simulink model "TosAeb"  (R2021a)
settings: Solver=FixedStepDiscrete  StartTime=0.0  StopTime=inf  FixedStep=0.01  RelTol=1e-3
undefined symbols (not defined in the file or in its folder): ACCModSts, AccSts1, AEB_ObjectMovingOrientation, AEB_ObjectMovingState, CN_BTCActive, CN_CurrentGearR, CN_CurrentGearR_DHT, CN_PTCActive, CN_VDCActive, CN_VSC_EspOff, CN_VSC_FCW_AEB_Error, CN_VSC_FCW_AEB_NoDispay, CN_VSC_FCW_AEB_PerfmDgdtn, CN_VSC_FCW_AEB_TempUnAvlbl, CN_VSC_VehStandstill, CNaccelAvoidMax, CNaccelAvoidMin, CNbrakePedalPress, CNcomfortStrLeftAccelMax, CNcomfortStrLeftAccelMin, CNcomfortStrRightAccelMax, CNcomfortStrRightAccelMin, CNDrvDoorOpen, CNDrvModeEco, CNDrvModeNorm, CNDrvModeOther, CNDrvModeSport, CNDrvSBRreminder, CNNull, CNobjInvalidID, CNPassDoorOpen, CNPassSBRreminder, CNPEBSAccelNegInf, CNPEBSAccelNull, CNPEBSTimeInf, CNPEBSTimeNull, CNSTOPMAX, CNSTOPNULL, CNsubjWidth, CNSwtOFF, CNSwtON, CNTTCMAX, CNTTCNULL, CNVehConfigAEBCycNone, CNVehConfigAEBNone, CNVehConfigAEBPedNone, CNVehConfigFCWNone, CNVehConfigJANone, CollisionSeverity, FALSE, FCWSnvty, JACycObjCalcInfoDefaultParams, JAPedObjCalcInfoDefaultParams, JAVehObjCalcInfoDefaultParams, latCycObjCalcInfoDefaultParams, latPedObjCalcInfoDefaultParams, longObjCalcInfoDefaultParams, ModSts, movingStatus, ObjectType ... (+1112)
libraries: simulink x3031, AEBSFunctionLib x50, Ta_Library x33, AEBSStateMachineLib x23, Mylibrary x12, simulink_extras x7, ObjSelect_lib x3, dspstat3 x2
(part 15 of 102)

== system TosAeb/FcwAebJa_Step_sys/ALG_AEBSystem/ALG_AEBController/AEB_FuncImpManagementApp/FuncImpArbCalc/BrkFuncImpArbCalc1 ==
  AEBModStsArb : Inport  OutDataTypeStr=Bus: BusAEBModStsArb
  FCTBModStsArb : Inport  Port=2  OutDataTypeStr=Enum: ModSts
  BrkFumcActiveCondCalc : Subsystem
  BrkFuncImpAborCondCalc : Subsystem
  BrkFuncImpArbCalc : Subsystem
  BrkFuncImpSuppCondCalc : Subsystem
  Bus Creator : BusCreator  Inputs=8
  Bus Creator1 : BusCreator  Inputs=8
  Bus Creator2 : BusCreator  Inputs=2
  Bus Creator3 : BusCreator  Inputs=8
  Bus Selector : BusSelector  OutputSignals=AEBpartFuncImpArb,cycAEBpartFuncImpArb,AEBfullFuncImpArb,JA_AEBfullFuncImpArb,cycAEBfullFuncImpArb,pedAEBfullFuncImpArb,EBAFuncImpArb,FCTBFuncImpArb
  brkFuncActvCond : Outport
  brkFuncImpArb : Outport  Port=2
  brkFuncImpSuppCondAbor : Outport  Port=3
  wires:
  BrkFumcActiveCondCalc/1(brkFuncActvCond) -> brkFuncActvCond/1
  AEBModStsArb/1 -> BrkFuncImpArbCalc/1(AEBModStsArb), BrkFuncImpAborCondCalc/1(AEBModStsArb), BrkFuncImpSuppCondCalc/1(AEBModStsArb)
  Bus Creator/1 -> Bus Creator2/1, BrkFuncImpArbCalc/2(brkFuncImpAborCondCond)  "brkFuncImpAborCond"
  BrkFuncImpArbCalc/2(cycAEBpartFuncImpArb) -> Bus Creator3/2  "cycAEBpartFuncImpArb"
  BrkFuncImpArbCalc/7(EBAFuncImpArb) -> Bus Creator3/7  "EBAFuncImpArb"
  BrkFuncImpArbCalc/4(JA_vehAEBfullFuncImpArb) -> Bus Creator3/4  "JA_AEBfullFuncImpArb"
  BrkFuncImpArbCalc/1(AEBpartFuncImpArb) -> Bus Creator3/1  "AEBpartFuncImpArb"
  BrkFuncImpArbCalc/5(cycAEBfullFuncImpArb) -> Bus Creator3/5  "cycAEBfullFuncImpArb"
  BrkFuncImpArbCalc/6(pedAEBfullFuncImpArb) -> Bus Creator3/6  "pedAEBfullFuncImpArb"
  BrkFuncImpArbCalc/8(FCTBFuncImpArb) -> Bus Creator3/8  "FCTBFuncImpArb"
  BrkFuncImpArbCalc/3(AEBfullFuncImpArb) -> Bus Creator3/3  "AEBfullFuncImpArb"
  Bus Selector/1 -> BrkFumcActiveCondCalc/1(AEBpartFuncImpArb)  "<AEBpartFuncImpArb>"
  Bus Selector/4 -> BrkFumcActiveCondCalc/4(JA_vehAEBfullFuncImpArb)  "<JA_AEBfullFuncImpArb>"
  Bus Selector/8 -> BrkFumcActiveCondCalc/8(FCTBFuncImpArb)  "<FCTBFuncImpArb>"
  Bus Selector/5 -> BrkFumcActiveCondCalc/5(cycAEBfullFuncImpArb)  "<cycAEBfullFuncImpArb>"
  Bus Selector/2 -> BrkFumcActiveCondCalc/2(cycAEBpartFuncImpArb)  "<cycAEBpartFuncImpArb>"
  Bus Selector/3 -> BrkFumcActiveCondCalc/3(AEBfullFuncImpArb)  "<AEBfullFuncImpArb>"
  Bus Selector/7 -> BrkFumcActiveCondCalc/7(EBAFuncImpArb)  "<EBAFuncImpArb>"
  Bus Selector/6 -> BrkFumcActiveCondCalc/6(pedAEBfullFuncImpArb)  "<pedAEBfullFuncImpArb>"
  FCTBModStsArb/1 -> BrkFuncImpArbCalc/3(FCTBModStsArb)
  BrkFuncImpSuppCondCalc/5(AEBfullFuncImpSuppCond) -> Bus Creator1/5  "vehAEBfullFuncImpSuppCond"
  BrkFuncImpSuppCondCalc/3(cycAEBpartFuncImpSuppCond) -> Bus Creator1/3  "cycAEBpartFuncImpSuppCond"
  BrkFuncImpSuppCondCalc/7(pedAEBfullFuncImpSuppCond) -> Bus Creator1/7  "pedAEBfullFuncImpSuppCond"
  BrkFuncImpSuppCondCalc/6(cycAEBfullFuncImpSuppCond) -> Bus Creator1/6  "cycAEBfullFuncImpSuppCond"
  BrkFuncImpSuppCondCalc/8(EBAFuncImpSuppCond) -> Bus Creator1/8  "vehEBAFuncImpSuppCond"
  BrkFuncImpSuppCondCalc/2(AEBpartFuncImpSuppCond) -> Bus Creator1/2  "vehAEBpartFuncImpSuppCond"
  BrkFuncImpSuppCondCalc/1(FCTBFuncImpSuppCond) -> Bus Creator1/1  "FCTBFuncImpSuppCond"
  BrkFuncImpSuppCondCalc/4(JA_vehAEBfullFuncImpSuppCond) -> Bus Creator1/4  "JA_vehAEBfullFuncImpSuppCond"
  Bus Creator1/1 -> Bus Creator2/2  "brkFuncImpSuppCond"
  Bus Creator2/1 -> brkFuncImpSuppCondAbor/1  "brkFuncImpSuppAbor"
  Bus Creator3/1 -> brkFuncImpArb/1, Bus Selector/1  "brkFuncImpArb"
  BrkFuncImpAborCondCalc/5(AEBfullFuncImpAborCond) -> Bus Creator/5  "vehAEBfullFuncImpAborCond"
  BrkFuncImpAborCondCalc/7(pedAEBfullFuncImpAborCond) -> Bus Creator/7  "pedAEBfullFuncImpAborCond"
  BrkFuncImpAborCondCalc/3(cycAEBpartFuncImpAborCond) -> Bus Creator/3  "cycAEBpartFuncImpAborCond"
  BrkFuncImpAborCondCalc/2(AEBpartFuncImpAborCond) -> Bus Creator/2  "vehAEBpartFuncImpAborCond"
  BrkFuncImpAborCondCalc/4(JA_vehAEBfullFuncImpAborCond) -> Bus Creator/4  "JA_vehAEBfullFuncImpAborCond"
  BrkFuncImpAborCondCalc/8(EBAFuncImpAborCond) -> Bus Creator/8  "vehEBAFuncImpAborCond"
  BrkFuncImpAborCondCalc/1(FCTBFuncImpAborCond) -> Bus Creator/1  "FCTBFuncImpAborCond"
  BrkFuncImpAborCondCalc/6(cycAEBfullFuncImpAborCond) -> Bus Creator/6  "cycAEBfullFuncImpAborCond"

== system TosAeb/FcwAebJa_Step_sys/ALG_AEBSystem/ALG_AEBController/AEB_FuncImpManagementApp/FuncImpArbCalc/BrkReadyFuncImpArbCalc ==
  prefillModStsArb : Inport
  ABAModStsArb : Inport  Port=2
  pedPrefillModStsArb : Inport  Port=3
  pedABAModStsArb : Inport  Port=4
  cycPrefillModStsArb : Inport  Port=5
  JA_vehPrefillModStsArb : Inport  Port=6
  JA_PTW_PrefillModStsArb : Inport  Port=7
  JA_cycPrefillModStsArb : Inport  Port=8
  JA_pedPrefillModStsArb : Inport  Port=9
  FCTB_PrefillModStsArb : Inport  Port=10
  FCTB_ABAModStsArb : Inport  Port=11
  brkFuncActiveCond : Inport  Port=12
  BrkFuncImpSuppCondCalc : Subsystem
  BrkReadyFuncActvCondCalc : Subsystem
  BrkReadyFuncImpAborCondCalc : Subsystem
  BrkReadyFuncImpArbCalc : Subsystem
  Bus Creator : BusCreator  Inputs=11
  Bus Creator1 : BusCreator  Inputs=11
  Bus Creator2 : BusCreator  Inputs=11
  Bus Creator3 : BusCreator  Inputs=2
  Bus Creator4 : BusCreator  Inputs=12
  Bus Selector : BusSelector  OutputSignals=prefillModStsArb,ABAModStsArb,pedPrefillModStsArb,pedABAModStsArb,cycPrefillModStsArb,JA_vehPrefillModStsArb,JA_PTW_PrefillModStsArb,JA_cycPrefillModStsArb,JA_pedPrefillModStsArb,brkFuncActiveCond
  Bus Selector1 : BusSelector  OutputSignals=prefillFuncImpArb,ABAFuncImpArb,pedPrefillFuncImpArb,pedABAFuncImpArb,cycPrefillFuncImpArb,JA_vehPrefillFuncImpArb,JA_PTW_PrefillFuncImpArb,JA_cycPrefillFuncImpArb,JA_pedPrefillFuncImpArb,FCTB_PrefillFuncImpArb,FCTB_ABAFuncImpArb
  Bus Selector2 : BusSelector  OutputSignals=prefillModStsArb,ABAModStsArb,pedABAModStsArb,pedPrefillModStsArb,cycPrefillModStsArb,JA_vehPrefillModStsArb,JA_PTW_PrefillModStsArb,JA_cycPrefillModStsArb,JA_pedPrefillModStsArb,brkFuncActiveCond
  Bus Selector3 : BusSelector  OutputSignals=prefillModStsArb,ABAModStsArb,pedPrefillModStsArb,pedABAModStsArb,cycPrefillModStsArb,JA_vehPrefillModStsArb,JA_PTW_PrefillModStsArb,JA_cycPrefillModStsArb,JA_pedPrefillModStsArb,FCTB_PrefillModStsArb,FCTB_ABAModStsArb
  Bus Selector4 : BusSelector  OutputSignals=cycPrefillFuncImpAborCond,pedPrefillFuncImpAborCond,vehPrefillFuncImpAborCond,vehABAFuncImpAborCond,pedABAFuncImpAborCond,JA_vehPrefillFuncImpAborCond,JA_pedPrefillFuncImpAborCond,JA_cycPrefillFuncImpAborCond,JA_PTW_PrefillFuncImpAborCond,FCTB_PrefillFuncImpAborCond,FCTB_ABAFuncImpAborCond
  brkReadyFuncActvCond : Outport
  brkReadyFuncImpArb : Outport  Port=2
  brkReadyFuncImpSuppCondAbor : Outport  Port=3
  wires:
  Bus Selector2/3 -> BrkFuncImpSuppCondCalc/3(pedABAModStsArb)  "<pedABAModStsArb>"
  Bus Selector1/11 -> BrkReadyFuncActvCondCalc/11(FCTB_ABAFuncImpArb)  "<FCTB_ABAFuncImpArb>"
  Bus Selector1/10 -> BrkReadyFuncActvCondCalc/10(FCTB_PrefillFuncImpArb)  "<FCTB_PrefillFuncImpArb>"
  Bus Selector1/9 -> BrkReadyFuncActvCondCalc/9(JA_pedPrefillFuncImpArb)  "<JA_pedPrefillFuncImpArb>"
  Bus Selector1/8 -> BrkReadyFuncActvCondCalc/8(JA_cycPrefillFuncImpArb)  "<JA_cycPrefillFuncImpArb>"
  Bus Selector1/7 -> BrkReadyFuncActvCondCalc/7(JA_PTW_PrefillFuncImpArb)  "<JA_PTW_PrefillFuncImpArb>"
  BrkReadyFuncImpArbCalc/11(FCTB_ABAFuncImpArb) -> Bus Creator1/11  "FCTB_ABAFuncImpArb"
  BrkReadyFuncImpArbCalc/10(FCTB_PrefillFuncImpArb) -> Bus Creator1/10  "FCTB_PrefillFuncImpArb"
  BrkReadyFuncImpArbCalc/9(JA_pedPrefillFuncImpArb) -> Bus Creator1/9  "JA_pedPrefillFuncImpArb"
  BrkReadyFuncImpArbCalc/8(JA_cycPrefillFuncImpArb) -> Bus Creator1/8  "JA_cycPrefillFuncImpArb"
  BrkReadyFuncImpArbCalc/7(JA_PTW_PrefillFuncImpArb) -> Bus Creator1/7  "JA_PTW_PrefillFuncImpArb"
  Bus Selector4/11 -> BrkReadyFuncImpArbCalc/22(FCTB_ABAFuncImpAborArb)  "<FCTB_ABAFuncImpAborCond>"
  Bus Selector4/10 -> BrkReadyFuncImpArbCalc/21(FCTB_PrefillFuncImpAborArb)  "<FCTB_PrefillFuncImpAborCond>"
  Bus Selector4/9 -> BrkReadyFuncImpArbCalc/20(JA_PTW_PrefillFuncImpAborArb)  "<JA_PTW_PrefillFuncImpAborCond>"
  Bus Selector4/8 -> BrkReadyFuncImpArbCalc/19(JA_cycPrefillFuncImpAborArb)  "<JA_cycPrefillFuncImpAborCond>"
  Bus Selector4/7 -> BrkReadyFuncImpArbCalc/18(JA_pedPrefillFuncImpAborArb)  "<JA_pedPrefillFuncImpAborCond>"
  Bus Selector4/6 -> BrkReadyFuncImpArbCalc/17(JA_vehPrefillFuncImpAborArb)  "<JA_vehPrefillFuncImpAborCond>"
  Bus Selector4/5 -> BrkReadyFuncImpArbCalc/16(pedABAFuncImpAborCond)  "<pedABAFuncImpAborCond>"
  Bus Selector4/1 -> BrkReadyFuncImpArbCalc/12(cycPrefillFuncImpAborCond)  "<cycPrefillFuncImpAborCond>"
  Bus Selector4/4 -> BrkReadyFuncImpArbCalc/15(vehABAFuncImpAborCond)  "<vehABAFuncImpAborCond>"
  Bus Selector4/2 -> BrkReadyFuncImpArbCalc/13(pedPrefillFuncImpAborCond)  "<pedPrefillFuncImpAborCond>"
  Bus Selector4/3 -> BrkReadyFuncImpArbCalc/14(vehPrefillFuncImpAborCond)  "<vehPrefillFuncImpAborCond>"
  Bus Selector3/11 -> BrkReadyFuncImpArbCalc/11(FCTB_ABAModStsArb)  "<FCTB_ABAModStsArb>"
  Bus Selector3/10 -> BrkReadyFuncImpArbCalc/10(FCTB_PrefillModStsArb)  "<FCTB_PrefillModStsArb>"
  Bus Selector3/1 -> BrkReadyFuncImpArbCalc/1(prefillModStsArb)  "<prefillModStsArb>"
  Bus Selector3/2 -> BrkReadyFuncImpArbCalc/2(ABAModStsArb)  "<ABAModStsArb>"
  Bus Selector3/3 -> BrkReadyFuncImpArbCalc/3(pedPrefillModStsArb)  "<pedPrefillModStsArb>"
  Bus Selector3/4 -> BrkReadyFuncImpArbCalc/4(pedABAModStsArb)  "<pedABAModStsArb>"
  Bus Selector3/5 -> BrkReadyFuncImpArbCalc/5(cycPrefillModStsArb)  "<cycPrefillModStsArb>"
  Bus Selector3/6 -> BrkReadyFuncImpArbCalc/6(JA_vehPrefillModStsArb)  "<JA_vehPrefillModStsArb>"
  Bus Selector3/7 -> BrkReadyFuncImpArbCalc/7(JA_PTW_PrefillModStsArb)  "<JA_PTW_PrefillModStsArb>"
  Bus Selector3/8 -> BrkReadyFuncImpArbCalc/8(JA_cycPrefillModStsArb)  "<JA_cycPrefillModStsArb>"
  Bus Selector3/9 -> BrkReadyFuncImpArbCalc/9(JA_pedPrefillModStsArb)  "<JA_pedPrefillModStsArb>"
  FCTB_ABAModStsArb/1 -> Bus Creator4/11  "FCTB_ABAModStsArb"
  FCTB_PrefillModStsArb/1 -> Bus Creator4/10  "FCTB_PrefillModStsArb"
  BrkFuncImpSuppCondCalc/11(FCTB_ABAFuncImpSuppCond) -> Bus Creator2/11  "FCTB_ABAFuncImpSuppCond"
  BrkFuncImpSuppCondCalc/10(FCTB_PrefillFuncImpSuppCond) -> Bus Creator2/10  "FCTB_PrefillFuncImpSuppCond"
  BrkFuncImpSuppCondCalc/9(JA_PTW_PrefillFuncImpSuppCond) -> Bus Creator2/9  "JA_PTW_PrefillFuncImpSuppCond"
  BrkFuncImpSuppCondCalc/8(JA_cycPrefillFuncImpSuppCond) -> Bus Creator2/8  "JA_cycPrefillFuncImpSuppCond"
  BrkFuncImpSuppCondCalc/7(JA_pedPrefillFuncImpSuppCond) -> Bus Creator2/7  "JA_pedPrefillFuncImpSuppCond"
  BrkFuncImpSuppCondCalc/6(JA_vehPrefillFuncImpSuppCond) -> Bus Creator2/6  "JA_vehPrefillFuncImpSuppCond"
  BrkReadyFuncImpAborCondCalc/11(FCTB_ABAFuncImpAborCond) -> Bus Creator/11  "FCTB_ABAFuncImpAborCond"
  BrkReadyFuncImpAborCondCalc/10(FCTB_PrefillFuncImpAborCond) -> Bus Creator/10  "FCTB_PrefillFuncImpAborCond"
  BrkReadyFuncImpAborCondCalc/9(JA_PTW_PrefillFuncImpAborCond) -> Bus Creator/9  "JA_PTW_PrefillFuncImpAborCond"
  BrkReadyFuncImpAborCondCalc/8(JA_cycPrefillFuncImpAborCond) -> Bus Creator/8  "JA_cycPrefillFuncImpAborCond"
  BrkReadyFuncImpAborCondCalc/7(JA_pedPrefillFuncImpAborCond) -> Bus Creator/7  "JA_pedPrefillFuncImpAborCond"
  BrkReadyFuncImpAborCondCalc/6(JA_vehPrefillFuncImpAborCond) -> Bus Creator/6  "JA_vehPrefillFuncImpAborCond"
  Bus Selector/4 -> BrkReadyFuncImpAborCondCalc/4(pedABAModStsArb)  "<pedABAModStsArb>"
  pedABAModStsArb/1 -> Bus Creator4/4  "pedABAModStsArb"
  Bus Selector2/10 -> BrkFuncImpSuppCondCalc/10(brkFuncActiveCond)  "<brkFuncActiveCond>"
  Bus Selector2/1 -> BrkFuncImpSuppCondCalc/1(prefillModStsArb)  "<prefillModStsArb>"
  Bus Selector2/2 -> BrkFuncImpSuppCondCalc/2(ABAModStsArb)  "<ABAModStsArb>"
  Bus Selector2/4 -> BrkFuncImpSuppCondCalc/4(pedPrefillModStsArb)  "<pedPrefillModStsArb>"
  Bus Selector2/5 -> BrkFuncImpSuppCondCalc/5(cycPrefillModStsArb)  "<cycPrefillModStsArb>"
  Bus Selector2/6 -> BrkFuncImpSuppCondCalc/6(JA_vehPrefillModStsArb)  "<JA_vehPrefillModStsArb>"
  Bus Selector2/7 -> BrkFuncImpSuppCondCalc/7(JA_PTW_PrefillModStsArb)  "<JA_PTW_PrefillModStsArb>"
  Bus Selector2/8 -> BrkFuncImpSuppCondCalc/8(JA_cycPrefillModStsArb)  "<JA_cycPrefillModStsArb>"
  Bus Selector2/9 -> BrkFuncImpSuppCondCalc/9(JA_pedPrefillModStsArb)  "<JA_pedPrefillModStsArb>"
  JA_cycPrefillModStsArb/1 -> Bus Creator4/8  "JA_cycPrefillModStsArb"
  cycPrefillModStsArb/1 -> Bus Creator4/5  "cycPrefillModStsArb"
  brkFuncActiveCond/1 -> Bus Creator4/12  "brkFuncActiveCond"
  pedPrefillModStsArb/1 -> Bus Creator4/3  "pedPrefillModStsArb"
  JA_vehPrefillModStsArb/1 -> Bus Creator4/6  "JA_vehPrefillModStsArb"
  ABAModStsArb/1 -> Bus Creator4/2  "ABAModStsArb"
  prefillModStsArb/1 -> Bus Creator4/1  "prefillModStsArb"
  JA_PTW_PrefillModStsArb/1 -> Bus Creator4/7  "JA_PTW_PrefillModStsArb"
  JA_pedPrefillModStsArb/1 -> Bus Creator4/9  "JA_pedPrefillModStsArb"
  Bus Selector/9 -> BrkReadyFuncImpAborCondCalc/9(JA_pedPrefillModStsArb)  "<JA_pedPrefillModStsArb>"
  Bus Selector/8 -> BrkReadyFuncImpAborCondCalc/8(JA_cycPrefillModStsArb)  "<JA_cycPrefillModStsArb>"
  Bus Selector/7 -> BrkReadyFuncImpAborCondCalc/7(JA_PTW_PrefillModStsArb)  "<JA_PTW_PrefillModStsArb>"
  Bus Creator3/1 -> brkReadyFuncImpSuppCondAbor/1
  Bus Creator2/1 -> Bus Creator3/2  "brkReadyFuncImpSuppCond"
  BrkFuncImpSuppCondCalc/1(cycPrefillFuncImpSuppCond) -> Bus Creator2/1  "cycPrefillFuncImpSuppCond"
  BrkFuncImpSuppCondCalc/2(pedPrefillFuncImpSuppCond) -> Bus Creator2/2  "pedPrefillFuncImpSuppCond"
  BrkFuncImpSuppCondCalc/3(prefillFuncImpSuppCond) -> Bus Creator2/3  "vehPrefillFuncImpSuppCond"
  BrkFuncImpSuppCondCalc/4(ABAFuncImpSuppCond) -> Bus Creator2/4  "vehABAFuncImpSuppCond"
  BrkFuncImpSuppCondCalc/5(pedABAFuncImpSuppCond) -> Bus Creator2/5  "pedABAFuncImpSuppCond"
  BrkReadyFuncActvCondCalc/1(brkReadyActvCond) -> brkReadyFuncActvCond/1
  Bus Selector1/6 -> BrkReadyFuncActvCondCalc/6(JA_vehPrefillFuncImpArb)  "<JA_vehPrefillFuncImpArb>"
  Bus Selector1/5 -> BrkReadyFuncActvCondCalc/5(cycPrefillFuncImpArb)  "<cycPrefillFuncImpArb>"
  Bus Selector1/4 -> BrkReadyFuncActvCondCalc/4(pedABAFuncImpArb)  "<pedABAFuncImpArb>"
  Bus Selector1/3 -> BrkReadyFuncActvCondCalc/3(pedPrefillFuncImpArb)  "<pedPrefillFuncImpArb>"
  Bus Selector1/2 -> BrkReadyFuncActvCondCalc/2(ABAFuncImpArb)  "<ABAFuncImpArb>"
  Bus Selector1/1 -> BrkReadyFuncActvCondCalc/1(PrefillFuncImpArb)  "<prefillFuncImpArb>"
  BrkReadyFuncImpArbCalc/1(prefillFuncImpArb) -> Bus Creator1/1  "prefillFuncImpArb"
  BrkReadyFuncImpArbCalc/6(JA_vehPrefillFuncImpArb) -> Bus Creator1/6  "JA_vehPrefillFuncImpArb"
  BrkReadyFuncImpArbCalc/2(ABAFuncImpArb) -> Bus Creator1/2  "ABAFuncImpArb"
  BrkReadyFuncImpArbCalc/5(cycPrefillFuncImpArb) -> Bus Creator1/5  "cycPrefillFuncImpArb"
  BrkReadyFuncImpArbCalc/3(pedPrefillFuncImpArb) -> Bus Creator1/3  "pedPrefillFuncImpArb"
  BrkReadyFuncImpArbCalc/4(pedABAFuncImpArb) -> Bus Creator1/4  "pedABAFuncImpArb"
  Bus Creator1/1 -> Bus Selector1/1, brkReadyFuncImpArb/1  "brkReadyFuncImpArb"
  Bus Creator/1 -> Bus Selector4/1, Bus Creator3/1  "brkReadyFuncImpAborCond"
  BrkReadyFuncImpAborCondCalc/1(cycPrefillFuncImpAborCond) -> Bus Creator/1  "cycPrefillFuncImpAborCond"
  BrkReadyFuncImpAborCondCalc/2(pedPrefillFuncImpAborCond) -> Bus Creator/2  "pedPrefillFuncImpAborCond"
  BrkReadyFuncImpAborCondCalc/3(prefillFuncImpAborCond) -> Bus Creator/3  "vehPrefillFuncImpAborCond"
  BrkReadyFuncImpAborCondCalc/4(ABAFuncImpAborCond) -> Bus Creator/4  "vehABAFuncImpAborCond"
  BrkReadyFuncImpAborCondCalc/5(pedABAFuncImpAborCond) -> Bus Creator/5  "pedABAFuncImpAborCond"
  Bus Selector/6 -> BrkReadyFuncImpAborCondCalc/6(JA_vehPrefillModStsArb)  "<JA_vehPrefillModStsArb>"
  Bus Selector/5 -> BrkReadyFuncImpAborCondCalc/5(cycPrefillModStsArb)  "<cycPrefillModStsArb>"
  Bus Selector/3 -> BrkReadyFuncImpAborCondCalc/3(pedPrefillModStsArb)  "<pedPrefillModStsArb>"
  Bus Selector/2 -> BrkReadyFuncImpAborCondCalc/2(ABAModStsArb)  "<ABAModStsArb>"
  Bus Selector/1 -> BrkReadyFuncImpAborCondCalc/1(prefillModStsArb)  "<prefillModStsArb>"
  Bus Selector/10 -> BrkReadyFuncImpAborCondCalc/10(brkFuncActiveCond)  "<brkFuncActiveCond>"
  Bus Creator4/1 -> Bus Selector3/1, Bus Selector2/1, Bus Selector/1

== system TosAeb/FcwAebJa_Step_sys/ALG_AEBSystem/ALG_AEBController/AEB_FuncImpManagementApp/FuncImpArbCalc/BrkReadyFuncImpArbCalc1 ==
  prefillModStsArb : Inport
  ABAModStsArb : Inport  Port=2
  pedPrefillModStsArb : Inport  Port=3
  pedABAModStsArb : Inport  Port=4
  cycPrefillModStsArb : Inport  Port=5
  JA_vehPrefillModStsArb : Inport  Port=6
  JA_PTW_PrefillModStsArb : Inport  Port=7
  JA_cycPrefillModStsArb : Inport  Port=8
  JA_pedPrefillModStsArb : Inport  Port=9
  brkFuncActiveCond : Inport  Port=10
  BrkFuncImpSuppCondCalc : Subsystem
  BrkReadyFuncActvCondCalc : Subsystem
  BrkReadyFuncImpAborCondCalc : Subsystem
  BrkReadyFuncImpArbCalc : Subsystem
  Bus Creator : BusCreator  Inputs=12
  Bus Creator1 : BusCreator  Inputs=6
  Bus Creator2 : BusCreator  Inputs=12
  Bus Creator3 : BusCreator  Inputs=2
  Bus Creator4 : BusCreator  Inputs=10
  Bus Selector : BusSelector  OutputSignals=prefillModStsArb,ABAModStsArb,pedPrefillModStsArb,pedABAModStsArb,cycPrefillModStsArb,JA_vehPrefillModStsArb,JA_PTW_PrefillModStsArb,JA_cycPrefillModStsArb,JA_pedPrefillModStsArb,brkFuncActiveCond
  Bus Selector1 : BusSelector  OutputSignals=prefillFuncImpArb,ABAFuncImpArb,pedPrefillFuncImpArb,pedABAFuncImpArb,cycPrefillFuncImpArb,JA_vehPrefillFuncImpArb
  Bus Selector2 : BusSelector  OutputSignals=prefillModStsArb,ABAModStsArb,pedPrefillModStsArb,cycPrefillModStsArb,JA_vehPrefillModStsArb,JA_PTW_PrefillModStsArb,JA_cycPrefillModStsArb,JA_pedPrefillModStsArb,brkFuncActiveCond
  Bus Selector3 : BusSelector  OutputSignals=prefillModStsArb,ABAModStsArb,pedPrefillModStsArb,cycPrefillModStsArb,JA_vehPrefillModStsArb,JA_PTW_PrefillModStsArb,JA_cycPrefillModStsArb,JA_pedPrefillModStsArb
  Bus Selector4 : BusSelector  OutputSignals=JA_vehPrefillFuncImpAborCond,cycPrefillFuncImpAborCond,pedPrefillFuncImpAborCond,vehPrefillFuncImpAborCond,vehABAFuncImpAborCond,pedABAFuncImpAborCond,JA_vehPrefillFuncImpAborArb,JA_pedPrefillFuncImpAborArb,JA_cycPrefillFuncImpAborArb,JA_PTW_PrefillFuncImpAborArb,FCTB_PrefillFuncImpAborArb,FCTB_ABAPrefillFuncImpAborArb
  brkReadyFuncActvCond : Outport
  brkReadyFuncImpArb : Outport  Port=2
  brkReadyFuncImpSuppCondAbor : Outport  Port=3
  wires:
  Bus Creator4/1 -> Bus Selector3/1, Bus Selector/1, Bus Selector2/1
  Bus Selector/10 -> BrkReadyFuncImpAborCondCalc/10(brkFuncActiveCond)  "<brkFuncActiveCond>"
  Bus Selector/1 -> BrkReadyFuncImpAborCondCalc/1(prefillModStsArb)  "<prefillModStsArb>"
  Bus Selector/2 -> BrkReadyFuncImpAborCondCalc/2(ABAModStsArb)  "<ABAModStsArb>"
  Bus Selector/3 -> BrkReadyFuncImpAborCondCalc/3(pedPrefillModStsArb)  "<pedPrefillModStsArb>"
  Bus Selector/5 -> BrkReadyFuncImpAborCondCalc/5(cycPrefillModStsArb)  "<cycPrefillModStsArb>"
  Bus Selector/6 -> BrkReadyFuncImpAborCondCalc/6(JA_vehPrefillModStsArb)  "<JA_vehPrefillModStsArb>"
  BrkReadyFuncImpAborCondCalc/6(pedABAFuncImpAborCond) -> Bus Creator/6  "pedABAFuncImpAborCond"
  BrkReadyFuncImpAborCondCalc/5(ABAFuncImpAborCond) -> Bus Creator/5  "vehABAFuncImpAborCond"
  BrkReadyFuncImpAborCondCalc/4(prefillFuncImpAborCond) -> Bus Creator/4  "vehPrefillFuncImpAborCond"
  BrkReadyFuncImpAborCondCalc/3(pedPrefillFuncImpAborCond) -> Bus Creator/3  "pedPrefillFuncImpAborCond"
  BrkReadyFuncImpAborCondCalc/2(cycPrefillFuncImpAborCond) -> Bus Creator/2  "cycPrefillFuncImpAborCond"
  BrkReadyFuncImpAborCondCalc/1(JA_vehPrefillFuncImpAborCond) -> Bus Creator/1  "JA_vehPrefillFuncImpAborCond"
  Bus Creator/1 -> Bus Selector4/1, Bus Creator3/1  "brkReadyFuncImpAborCond"
  Bus Creator1/1 -> Bus Selector1/1, brkReadyFuncImpArb/1  "brkReadyFuncImpArb"
  BrkReadyFuncImpArbCalc/4(pedABAFuncImpArb) -> Bus Creator1/4  "pedABAFuncImpArb"
  BrkReadyFuncImpArbCalc/3(pedPrefillFuncImpArb) -> Bus Creator1/3  "pedPrefillFuncImpArb"
  BrkReadyFuncImpArbCalc/5(cycPrefillFuncImpArb) -> Bus Creator1/5  "cycPrefillFuncImpArb"
  BrkReadyFuncImpArbCalc/2(ABAFuncImpArb) -> Bus Creator1/2  "ABAFuncImpArb"
  BrkReadyFuncImpArbCalc/6(JA_vehPrefillFuncImpArb) -> Bus Creator1/6  "JA_vehPrefillFuncImpArb"
  BrkReadyFuncImpArbCalc/1(prefillFuncImpArb) -> Bus Creator1/1  "prefillFuncImpArb"
  Bus Selector1/1 -> BrkReadyFuncActvCondCalc/1(PrefillFuncImpArb)  "<prefillFuncImpArb>"
  Bus Selector1/2 -> BrkReadyFuncActvCondCalc/2(ABAFuncImpArb)  "<ABAFuncImpArb>"
  Bus Selector1/3 -> BrkReadyFuncActvCondCalc/3(pedPrefillFuncImpArb)  "<pedPrefillFuncImpArb>"
  Bus Selector1/4 -> BrkReadyFuncActvCondCalc/4(pedABAFuncImpArb)  "<pedABAFuncImpArb>"
  Bus Selector1/5 -> BrkReadyFuncActvCondCalc/5(cycPrefillFuncImpArb)  "<cycPrefillFuncImpArb>"
  Bus Selector1/6 -> BrkReadyFuncActvCondCalc/6(JA_vehPrefillFuncImpArb)  "<JA_vehPrefillFuncImpArb>"
  BrkReadyFuncActvCondCalc/1(brkReadyActvCond) -> brkReadyFuncActvCond/1
  BrkFuncImpSuppCondCalc/6(pedABAFuncImpSuppCond) -> Bus Creator2/6  "pedABAFuncImpSuppCond"
  BrkFuncImpSuppCondCalc/5(ABAFuncImpSuppCond) -> Bus Creator2/5  "vehABAFuncImpSuppCond"
  BrkFuncImpSuppCondCalc/4(prefillFuncImpSuppCond) -> Bus Creator2/4  "vehPrefillFuncImpSuppCond"
  BrkFuncImpSuppCondCalc/3(pedPrefillFuncImpSuppCond) -> Bus Creator2/3  "pedPrefillFuncImpSuppCond"
  BrkFuncImpSuppCondCalc/2(cycPrefillFuncImpSuppCond) -> Bus Creator2/2  "cycPrefillFuncImpSuppCond"
  BrkFuncImpSuppCondCalc/1(JA_PrefillFuncImpSuppCond) -> Bus Creator2/1  "JA_vehPrefillFuncImpSuppCond"
  Bus Creator2/1 -> Bus Creator3/2  "brkReadyFuncImpSuppCond"
  Bus Creator3/1 -> brkReadyFuncImpSuppCondAbor/1
  Bus Selector/7 -> BrkReadyFuncImpAborCondCalc/7(JA_PTW_PrefillModStsArb)  "<JA_PTW_PrefillModStsArb>"
  Bus Selector/8 -> BrkReadyFuncImpAborCondCalc/8(JA_cycPrefillModStsArb)  "<JA_cycPrefillModStsArb>"
  Bus Selector/9 -> BrkReadyFuncImpAborCondCalc/9(JA_pedPrefillModStsArb)  "<JA_pedPrefillModStsArb>"
  JA_pedPrefillModStsArb/1 -> Bus Creator4/9  "JA_pedPrefillModStsArb"
  JA_PTW_PrefillModStsArb/1 -> Bus Creator4/7  "JA_PTW_PrefillModStsArb"
  prefillModStsArb/1 -> Bus Creator4/1  "prefillModStsArb"
  ABAModStsArb/1 -> Bus Creator4/2  "ABAModStsArb"
  JA_vehPrefillModStsArb/1 -> Bus Creator4/6  "JA_vehPrefillModStsArb"
  pedPrefillModStsArb/1 -> Bus Creator4/3  "pedPrefillModStsArb"
  brkFuncActiveCond/1 -> Bus Creator4/10  "brkFuncActiveCond"
  cycPrefillModStsArb/1 -> Bus Creator4/5  "cycPrefillModStsArb"
  JA_cycPrefillModStsArb/1 -> Bus Creator4/8  "JA_cycPrefillModStsArb"
  Bus Selector2/8 -> BrkFuncImpSuppCondCalc/8(JA_pedPrefillModStsArb)  "<JA_pedPrefillModStsArb>"
  Bus Selector2/7 -> BrkFuncImpSuppCondCalc/7(JA_cycPrefillModStsArb)  "<JA_cycPrefillModStsArb>"
  Bus Selector2/6 -> BrkFuncImpSuppCondCalc/6(JA_PTW_PrefillModStsArb)  "<JA_PTW_PrefillModStsArb>"
  Bus Selector2/5 -> BrkFuncImpSuppCondCalc/5(JA_vehPrefillModStsArb)  "<JA_vehPrefillModStsArb>"
  Bus Selector2/4 -> BrkFuncImpSuppCondCalc/4(cycPrefillModStsArb)  "<cycPrefillModStsArb>"
  Bus Selector2/3 -> BrkFuncImpSuppCondCalc/3(pedPrefillModStsArb)  "<pedPrefillModStsArb>"
  Bus Selector2/2 -> BrkFuncImpSuppCondCalc/2(ABAModStsArb)  "<ABAModStsArb>"
  Bus Selector2/1 -> BrkFuncImpSuppCondCalc/1(prefillModStsArb)  "<prefillModStsArb>"